simulink library "inp"  (Simulink 8.2)
settings: Solver=ode45  SolverMode=SingleTasking  StartTime=0.0  StopTime=10.0  RelTol=1e-3
undefined symbols (not defined in the file or in its folder): C_TICK_TIME_S, ENUM_FAULTSTATE_FAIL, ENUM_FAULTSTATE_INDETERMINATE, ENUM_FAULTSTATE_PASS, ENUM_KEYSWITCH_ON, FALSE, KINP_ABS1CANCommuOk_flg_ovrdflg, KINP_ABS1CANCommuOk_flg_ovrdval, KINP_ABS1CANPeriodTime_s, KINP_ABS1CANRolCntOk_flg_ovrdflg, KINP_ABS1CANRolCntOk_flg_ovrdval, KINP_ABS2CANChkSumOk_flg_ovrdflg, KINP_ABS2CANChkSumOk_flg_ovrdval, KINP_ABS2CANCommuOk_flg_ovrdflg, KINP_ABS2CANCommuOk_flg_ovrdval, KINP_ABS2CANPeriodTime_s, KINP_ABS2CANRolCntOk_flg_ovrdflg, KINP_ABS2CANRolCntOk_flg_ovrdval, KINP_ABS3CANCommuOk_flg_ovrdflg, KINP_ABS3CANCommuOk_flg_ovrdval, KINP_ABS4CANCommuOk_flg_ovrdflg, KINP_ABS4CANCommuOk_flg_ovrdval, KINP_ABSActive_flg_defval, KINP_ABSActive_flg_invalid, KINP_ABSActive_flg_ovrdflg, KINP_ABSActive_flg_ovrdval, KINP_ABSActive_flg_setvalid, KINP_ABSActive_flg_srvcflg, KINP_ABSActive_flg_srvcval, KINP_ABSCANAlive_flg_ovrdflg, KINP_ABSCANAlive_flg_ovrdval, KINP_ABSCANCommuOk_flg_ovrdflg, KINP_ABSCANCommuOk_flg_ovrdval, KINP_ABSCANValidOk_flg_ovrdflg, KINP_ABSCANValidOk_flg_ovrdval, KINP_ABSFault_flg_defval, KINP_ABSFault_flg_invalid, KINP_ABSFault_flg_ovrdflg, KINP_ABSFault_flg_ovrdval, KINP_ABSFault_flg_setvalid, KINP_ABSFault_flg_srvcflg, KINP_ABSFault_flg_srvcval, KINP_AC1CANCommuOk_flg_ovrdflg, KINP_AC1CANCommuOk_flg_ovrdval, KINP_ACCANAlive_flg_ovrdflg, KINP_ACCANAlive_flg_ovrdval, KINP_ACCANCommuOk_flg_ovrdflg, KINP_ACCANCommuOk_flg_ovrdval, KINP_ACCANValidOk_flg_ovrdflg, KINP_ACCANValidOk_flg_ovrdval, KINP_ACCCancelH_flg_defval, KINP_ACCCancelH_flg_invalid, KINP_ACCCancelH_flg_ovrdflg, KINP_ACCCancelH_flg_ovrdval, KINP_ACCCancelH_flg_setvalid, KINP_ACCCancelH_flg_srvcflg, KINP_ACCCancelH_flg_srvcval, KINP_AccelPedalPosn_pct_defval, KINP_AccelPedalPosn_pct_gain, KINP_AccelPedalPosn_pct_invalid ... (+1866)
libraries: inp x192, hcu_lib x34, simulink x33
(part 11 of 21)

== system inp/INP/EPM_signals/Cam_Obstacle_8 ==
  BHAL_outputs : Inport
  CANvalid_bus : Inport  Port=2
  Bus Creator : BusCreator  Inputs=40
  CANVBusSel{141..150} (10 alike) : BusSelector  OutputSignals=VINP_CANMsgValidEPM_flg.VINP_CANMsgValidEPM27_flg
  CANVBusSel{151..155} (5 alike) : BusSelector  OutputSignals=VINP_CANMsgValidEPM_flg.VINP_CANMsgValidEPM28_flg
  CANVBusSel{156..160} (5 alike) : BusSelector  OutputSignals=VINP_CANMsgValidEPM_flg.VINP_CANMsgValidEPM29_flg
  Constant{141..160} (20 alike) : Constant  Value=TRUE
  Conv{141,145..149,153..154} (8 alike) : DataTypeConversion  OutDataTypeStr=uint8
  Conv{142..144,151..152,156..159} (9 alike) : DataTypeConversion  OutDataTypeStr=single
  Conv150 : DataTypeConversion  OutDataTypeStr=boolean
  Conv155 : DataTypeConversion  OutDataTypeStr=boolean
  Conv160 : DataTypeConversion  OutDataTypeStr=boolean
  HalBusSel141 : BusSelector  OutputSignals=VHAL_CamObs8ID_num
  HalBusSel142 : BusSelector  OutputSignals=VHAL_CamObs8PosX_m
  HalBusSel143 : BusSelector  OutputSignals=VHAL_CamObs8PosY_m
  HalBusSel144 : BusSelector  OutputSignals=VHAL_CamObs8VelX_mps
  HalBusSel145 : BusSelector  OutputSignals=VHAL_CamObs8Type_enum
  HalBusSel146 : BusSelector  OutputSignals=VHAL_CamObs8Status_enum
  HalBusSel147 : BusSelector  OutputSignals=VHAL_CamObs8Valid_enum
  HalBusSel148 : BusSelector  OutputSignals=VHAL_CamObs8MoveInO_enum
  HalBusSel149 : BusSelector  OutputSignals=VHAL_CamObs8Blinker_enum
  HalBusSel150 : BusSelector  OutputSignals=VHAL_CamObs8BrakeLight_flg
  HalBusSel151 : BusSelector  OutputSignals=VHAL_CamObs8Length_m
  HalBusSel152 : BusSelector  OutputSignals=VHAL_CamObs8Width_m
  HalBusSel153 : BusSelector  OutputSignals=VHAL_CamObs8Age_cnt
  HalBusSel154 : BusSelector  OutputSignals=VHAL_CamObs8Lane_enum
  HalBusSel155 : BusSelector  OutputSignals=VHAL_CamObs8CIPV_flg
  HalBusSel156 : BusSelector  OutputSignals=VHAL_CamObs8VelY_mps
  HalBusSel157 : BusSelector  OutputSignals=VHAL_CamObs8AccX_mps2
  HalBusSel158 : BusSelector  OutputSignals=VHAL_CamObs8Ang_deg
  HalBusSel159 : BusSelector  OutputSignals=VHAL_CamObs8AngRate_degps
  HalBusSel160 : BusSelector  OutputSignals=VHAL_CamObs8Replaced_flg
  Term{141..160} (20 alike) : Terminator
  Cam_Obstacle_8 : Outport
  wires:
  [BHAL_outputs] -> HalBusSel141/1
  HalBusSel141/1 -> Conv141/1  "<VHAL_CamObs8ID_num>"
  [CANvalid_bus] -> CANVBusSel141/1
  Conv141/1 -> Bus Creator/1  "VINP_CamObs8ID_num_InHAL"
  Constant141/1 -> Bus Creator/2  "VINP_CamObs8IDValid_flg_InHAL"
  CANVBusSel141/1 -> Term141/1  "<VINP_CANMsgValidEPM27_flg>"
  [BHAL_outputs] -> HalBusSel142/1
  HalBusSel142/1 -> Conv142/1  "<VHAL_CamObs8PosX_m>"
  [CANvalid_bus] -> CANVBusSel142/1
  Conv142/1 -> Bus Creator/3  "VINP_CamObs8PosX_m_InHAL"
  Constant142/1 -> Bus Creator/4  "VINP_CamObs8PosXValid_flg_InHAL"
  CANVBusSel142/1 -> Term142/1  "<VINP_CANMsgValidEPM27_flg>"
  [BHAL_outputs] -> HalBusSel143/1
  HalBusSel143/1 -> Conv143/1  "<VHAL_CamObs8PosY_m>"
  [CANvalid_bus] -> CANVBusSel143/1
  Conv143/1 -> Bus Creator/5  "VINP_CamObs8PosY_m_InHAL"
  Constant143/1 -> Bus Creator/6  "VINP_CamObs8PosYValid_flg_InHAL"
  CANVBusSel143/1 -> Term143/1  "<VINP_CANMsgValidEPM27_flg>"
  [BHAL_outputs] -> HalBusSel144/1
  HalBusSel144/1 -> Conv144/1  "<VHAL_CamObs8VelX_mps>"
  [CANvalid_bus] -> CANVBusSel144/1
  Conv144/1 -> Bus Creator/7  "VINP_CamObs8VelX_mps_InHAL"
  Constant144/1 -> Bus Creator/8  "VINP_CamObs8VelXValid_flg_InHAL"
  CANVBusSel144/1 -> Term144/1  "<VINP_CANMsgValidEPM27_flg>"
  [BHAL_outputs] -> HalBusSel145/1
  HalBusSel145/1 -> Conv145/1  "<VHAL_CamObs8Type_enum>"
  [CANvalid_bus] -> CANVBusSel145/1
  Conv145/1 -> Bus Creator/9  "VINP_CamObs8Type_enum_InHAL"
  Constant145/1 -> Bus Creator/10  "VINP_CamObs8TypeValid_flg_InHAL"
  CANVBusSel145/1 -> Term145/1  "<VINP_CANMsgValidEPM27_flg>"
  [BHAL_outputs] -> HalBusSel146/1
  Conv146/1 -> Bus Creator/11  "VINP_CamObs8Status_enum_InHAL"
  HalBusSel146/1 -> Conv146/1  "<VHAL_CamObs8Status_enum>"
  [CANvalid_bus] -> CANVBusSel146/1
  Constant146/1 -> Bus Creator/12  "VINP_CamObs8StatusValid_flg_InHAL"
  CANVBusSel146/1 -> Term146/1  "<VINP_CANMsgValidEPM27_flg>"
  [BHAL_outputs] -> HalBusSel147/1
  [CANvalid_bus] -> CANVBusSel147/1
  HalBusSel147/1 -> Conv147/1  "<VHAL_CamObs8Valid_enum>"
  Conv147/1 -> Bus Creator/13  "VINP_CamObs8Valid_enum_InHAL"
  Constant147/1 -> Bus Creator/14  "VINP_CamObs8ValidValid_flg_InHAL"
  CANVBusSel147/1 -> Term147/1  "<VINP_CANMsgValidEPM27_flg>"
  [BHAL_outputs] -> HalBusSel148/1
  Conv148/1 -> Bus Creator/15  "VINP_CamObs8MoveInO_enum_InHAL"
  HalBusSel148/1 -> Conv148/1  "<VHAL_CamObs8MoveInO_enum>"
  [CANvalid_bus] -> CANVBusSel148/1
  Constant148/1 -> Bus Creator/16  "VINP_CamObs8MoveInOValid_flg_InHAL"
  CANVBusSel148/1 -> Term148/1  "<VINP_CANMsgValidEPM27_flg>"
  [BHAL_outputs] -> HalBusSel149/1
  Conv149/1 -> Bus Creator/17  "VINP_CamObs8Blinker_enum_InHAL"
  HalBusSel149/1 -> Conv149/1  "<VHAL_CamObs8Blinker_enum>"
  [CANvalid_bus] -> CANVBusSel149/1
  Constant149/1 -> Bus Creator/18  "VINP_CamObs8BlinkerValid_flg_InHAL"
  CANVBusSel149/1 -> Term149/1  "<VINP_CANMsgValidEPM27_flg>"
  [BHAL_outputs] -> HalBusSel150/1
  Conv150/1 -> Bus Creator/19  "VINP_CamObs8BrakeLight_flg_InHAL"
  HalBusSel150/1 -> Conv150/1  "<VHAL_CamObs8BrakeLight_flg>"
  [CANvalid_bus] -> CANVBusSel150/1
  Constant150/1 -> Bus Creator/20  "VINP_CamObs8BrakeLightValid_flg_InHAL"
  CANVBusSel150/1 -> Term150/1  "<VINP_CANMsgValidEPM27_flg>"
  [BHAL_outputs] -> HalBusSel151/1
  HalBusSel151/1 -> Conv151/1  "<VHAL_CamObs8Length_m>"
  [CANvalid_bus] -> CANVBusSel151/1
  Conv151/1 -> Bus Creator/21  "VINP_CamObs8Length_m_InHAL"
  Constant151/1 -> Bus Creator/22  "VINP_CamObs8LengthValid_flg_InHAL"
  CANVBusSel151/1 -> Term151/1  "<VINP_CANMsgValidEPM28_flg>"
  [BHAL_outputs] -> HalBusSel152/1
  HalBusSel152/1 -> Conv152/1  "<VHAL_CamObs8Width_m>"
  [CANvalid_bus] -> CANVBusSel152/1
  Conv152/1 -> Bus Creator/23  "VINP_CamObs8Width_m_InHAL"
  Constant152/1 -> Bus Creator/24  "VINP_CamObs8WidthValid_flg_InHAL"
  CANVBusSel152/1 -> Term152/1  "<VINP_CANMsgValidEPM28_flg>"
  [BHAL_outputs] -> HalBusSel153/1
  HalBusSel153/1 -> Conv153/1  "<VHAL_CamObs8Age_cnt>"
  [CANvalid_bus] -> CANVBusSel153/1
  Conv153/1 -> Bus Creator/25  "VINP_CamObs8Age_cnt_InHAL"
  Constant153/1 -> Bus Creator/26  "VINP_CamObs8AgeValid_flg_InHAL"
  CANVBusSel153/1 -> Term153/1  "<VINP_CANMsgValidEPM28_flg>"
  [BHAL_outputs] -> HalBusSel154/1
  HalBusSel154/1 -> Conv154/1  "<VHAL_CamObs8Lane_enum>"
  [CANvalid_bus] -> CANVBusSel154/1
  Conv154/1 -> Bus Creator/27  "VINP_CamObs8Lane_enum_InHAL"
  Constant154/1 -> Bus Creator/28  "VINP_CamObs8LaneValid_flg_InHAL"
  CANVBusSel154/1 -> Term154/1  "<VINP_CANMsgValidEPM28_flg>"
  [BHAL_outputs] -> HalBusSel155/1
  HalBusSel155/1 -> Conv155/1  "<VHAL_CamObs8CIPV_flg>"
  [CANvalid_bus] -> CANVBusSel155/1
  Conv155/1 -> Bus Creator/29  "VINP_CamObs8CIPV_flg_InHAL"
  Constant155/1 -> Bus Creator/30  "VINP_CamObs8CIPVValid_flg_InHAL"
  CANVBusSel155/1 -> Term155/1  "<VINP_CANMsgValidEPM28_flg>"
  [BHAL_outputs] -> HalBusSel156/1
  HalBusSel156/1 -> Conv156/1  "<VHAL_CamObs8VelY_mps>"
  [CANvalid_bus] -> CANVBusSel156/1
  Conv156/1 -> Bus Creator/31  "VINP_CamObs8VelY_mps_InHAL"
  Constant156/1 -> Bus Creator/32  "VINP_CamObs8VelYValid_flg_InHAL"
  CANVBusSel156/1 -> Term156/1  "<VINP_CANMsgValidEPM29_flg>"
  [BHAL_outputs] -> HalBusSel157/1
  HalBusSel157/1 -> Conv157/1  "<VHAL_CamObs8AccX_mps2>"
  [CANvalid_bus] -> CANVBusSel157/1
  Conv157/1 -> Bus Creator/33  "VINP_CamObs8AccX_mps2_InHAL"
  Constant157/1 -> Bus Creator/34  "VINP_CamObs8AccXValid_flg_InHAL"
  CANVBusSel157/1 -> Term157/1  "<VINP_CANMsgValidEPM29_flg>"
  [BHAL_outputs] -> HalBusSel158/1
  HalBusSel158/1 -> Conv158/1  "<VHAL_CamObs8Ang_deg>"
  [CANvalid_bus] -> CANVBusSel158/1
  Conv158/1 -> Bus Creator/35  "VINP_CamObs8Ang_deg_InHAL"
  Constant158/1 -> Bus Creator/36  "VINP_CamObs8AngValid_flg_InHAL"
  CANVBusSel158/1 -> Term158/1  "<VINP_CANMsgValidEPM29_flg>"
  [BHAL_outputs] -> HalBusSel159/1
  Conv159/1 -> Bus Creator/37  "VINP_CamObs8AngRate_degps_InHAL"
  HalBusSel159/1 -> Conv159/1  "<VHAL_CamObs8AngRate_degps>"
  [CANvalid_bus] -> CANVBusSel159/1
  Constant159/1 -> Bus Creator/38  "VINP_CamObs8AngRateValid_flg_InHAL"
  CANVBusSel159/1 -> Term159/1  "<VINP_CANMsgValidEPM29_flg>"
  [BHAL_outputs] -> HalBusSel160/1
  Conv160/1 -> Bus Creator/39  "VINP_CamObs8Replaced_flg_InHAL"
  HalBusSel160/1 -> Conv160/1  "<VHAL_CamObs8Replaced_flg>"
  [CANvalid_bus] -> CANVBusSel160/1
  Constant160/1 -> Bus Creator/40  "VINP_CamObs8ReplacedValid_flg_InHAL"
  CANVBusSel160/1 -> Term160/1  "<VINP_CANMsgValidEPM29_flg>"
  BHAL_outputs/1 -> [BHAL_outputs]
  CANvalid_bus/1 -> [CANvalid_bus]
  Bus Creator/1 -> Cam_Obstacle_8/1  "VINP_Obstacle8_BUS_InHAL"

== system inp/INP/EPM_signals/Cam_Obstacle_9 ==
  BHAL_outputs : Inport
  CANvalid_bus : Inport  Port=2
  Bus Creator : BusCreator  Inputs=40
  CANVBusSel{161..170} (10 alike) : BusSelector  OutputSignals=VINP_CANMsgValidEPM_flg.VINP_CANMsgValidEPM30_flg
  CANVBusSel{171..175} (5 alike) : BusSelector  OutputSignals=VINP_CANMsgValidEPM_flg.VINP_CANMsgValidEPM31_flg
  CANVBusSel{176..180} (5 alike) : BusSelector  OutputSignals=VINP_CANMsgValidEPM_flg.VINP_CANMsgValidEPM32_flg
  Constant{161..180} (20 alike) : Constant  Value=TRUE
  Conv{161,165..169,173..174} (8 alike) : DataTypeConversion  OutDataTypeStr=uint8
  Conv{162..164,171..172,176..179} (9 alike) : DataTypeConversion  OutDataTypeStr=single
  Conv170 : DataTypeConversion  OutDataTypeStr=boolean
  Conv175 : DataTypeConversion  OutDataTypeStr=boolean
  Conv180 : DataTypeConversion  OutDataTypeStr=boolean
  HalBusSel161 : BusSelector  OutputSignals=VHAL_CamObs9ID_num
  HalBusSel162 : BusSelector  OutputSignals=VHAL_CamObs9PosX_m
  HalBusSel163 : BusSelector  OutputSignals=VHAL_CamObs9PosY_m
  HalBusSel164 : BusSelector  OutputSignals=VHAL_CamObs9VelX_mps
  HalBusSel165 : BusSelector  OutputSignals=VHAL_CamObs9Type_enum
  HalBusSel166 : BusSelector  OutputSignals=VHAL_CamObs9Status_enum
  HalBusSel167 : BusSelector  OutputSignals=VHAL_CamObs9Valid_enum
  HalBusSel168 : BusSelector  OutputSignals=VHAL_CamObs9MoveInO_enum
  HalBusSel169 : BusSelector  OutputSignals=VHAL_CamObs9Blinker_enum
  HalBusSel170 : BusSelector  OutputSignals=VHAL_CamObs9BrakeLight_flg
  HalBusSel171 : BusSelector  OutputSignals=VHAL_CamObs9Length_m
  HalBusSel172 : BusSelector  OutputSignals=VHAL_CamObs9Width_m
  HalBusSel173 : BusSelector  OutputSignals=VHAL_CamObs9Age_cnt
  HalBusSel174 : BusSelector  OutputSignals=VHAL_CamObs9Lane_enum
  HalBusSel175 : BusSelector  OutputSignals=VHAL_CamObs9CIPV_flg
  HalBusSel176 : BusSelector  OutputSignals=VHAL_CamObs9VelY_mps
  HalBusSel177 : BusSelector  OutputSignals=VHAL_CamObs9AccX_mps2
  HalBusSel178 : BusSelector  OutputSignals=VHAL_CamObs9Ang_deg
  HalBusSel179 : BusSelector  OutputSignals=VHAL_CamObs9AngRate_degps
  HalBusSel180 : BusSelector  OutputSignals=VHAL_CamObs9Replaced_flg
  Term{161..180} (20 alike) : Terminator
  Cam_Obstacle_9 : Outport
  wires:
  [BHAL_outputs] -> HalBusSel161/1
  HalBusSel161/1 -> Conv161/1  "<VHAL_CamObs9ID_num>"
  [CANvalid_bus] -> CANVBusSel161/1
  Conv161/1 -> Bus Creator/1  "VINP_CamObs9ID_num_InHAL"
  Constant161/1 -> Bus Creator/2  "VINP_CamObs9IDValid_flg_InHAL"
  CANVBusSel161/1 -> Term161/1  "<VINP_CANMsgValidEPM30_flg>"
  [BHAL_outputs] -> HalBusSel162/1
  HalBusSel162/1 -> Conv162/1  "<VHAL_CamObs9PosX_m>"
  [CANvalid_bus] -> CANVBusSel162/1
  Conv162/1 -> Bus Creator/3  "VINP_CamObs9PosX_m_InHAL"
  Constant162/1 -> Bus Creator/4  "VINP_CamObs9PosXValid_flg_InHAL"
  CANVBusSel162/1 -> Term162/1  "<VINP_CANMsgValidEPM30_flg>"
  [BHAL_outputs] -> HalBusSel163/1
  HalBusSel163/1 -> Conv163/1  "<VHAL_CamObs9PosY_m>"
  [CANvalid_bus] -> CANVBusSel163/1
  Conv163/1 -> Bus Creator/5  "VINP_CamObs9PosY_m_InHAL"
  Constant163/1 -> Bus Creator/6  "VINP_CamObs9PosYValid_flg_InHAL"
  CANVBusSel163/1 -> Term163/1  "<VINP_CANMsgValidEPM30_flg>"
  [BHAL_outputs] -> HalBusSel164/1
  HalBusSel164/1 -> Conv164/1  "<VHAL_CamObs9VelX_mps>"
  [CANvalid_bus] -> CANVBusSel164/1
  Conv164/1 -> Bus Creator/7  "VINP_CamObs9VelX_mps_InHAL"
  Constant164/1 -> Bus Creator/8  "VINP_CamObs9VelXValid_flg_InHAL"
  CANVBusSel164/1 -> Term164/1  "<VINP_CANMsgValidEPM30_flg>"
  [BHAL_outputs] -> HalBusSel165/1
  HalBusSel165/1 -> Conv165/1  "<VHAL_CamObs9Type_enum>"
  [CANvalid_bus] -> CANVBusSel165/1
  Conv165/1 -> Bus Creator/9  "VINP_CamObs9Type_enum_InHAL"
  Constant165/1 -> Bus Creator/10  "VINP_CamObs9TypeValid_flg_InHAL"
  CANVBusSel165/1 -> Term165/1  "<VINP_CANMsgValidEPM30_flg>"
  [BHAL_outputs] -> HalBusSel166/1
  Conv166/1 -> Bus Creator/11  "VINP_CamObs9Status_enum_InHAL"
  HalBusSel166/1 -> Conv166/1  "<VHAL_CamObs9Status_enum>"
  [CANvalid_bus] -> CANVBusSel166/1
  Constant166/1 -> Bus Creator/12  "VINP_CamObs9StatusValid_flg_InHAL"
  CANVBusSel166/1 -> Term166/1  "<VINP_CANMsgValidEPM30_flg>"
  [BHAL_outputs] -> HalBusSel167/1
  [CANvalid_bus] -> CANVBusSel167/1
  HalBusSel167/1 -> Conv167/1  "<VHAL_CamObs9Valid_enum>"
  Conv167/1 -> Bus Creator/13  "VINP_CamObs9Valid_enum_InHAL"
  Constant167/1 -> Bus Creator/14  "VINP_CamObs9ValidValid_flg_InHAL"
  CANVBusSel167/1 -> Term167/1  "<VINP_CANMsgValidEPM30_flg>"
  [BHAL_outputs] -> HalBusSel168/1
  Conv168/1 -> Bus Creator/15  "VINP_CamObs9MoveInO_enum_InHAL"
  HalBusSel168/1 -> Conv168/1  "<VHAL_CamObs9MoveInO_enum>"
  [CANvalid_bus] -> CANVBusSel168/1
  Constant168/1 -> Bus Creator/16  "VINP_CamObs9MoveInOValid_flg_InHAL"
  CANVBusSel168/1 -> Term168/1  "<VINP_CANMsgValidEPM30_flg>"
  [BHAL_outputs] -> HalBusSel169/1
  Conv169/1 -> Bus Creator/17  "VINP_CamObs9Blinker_enum_InHAL"
  HalBusSel169/1 -> Conv169/1  "<VHAL_CamObs9Blinker_enum>"
  [CANvalid_bus] -> CANVBusSel169/1
  Constant169/1 -> Bus Creator/18  "VINP_CamObs9BlinkerValid_flg_InHAL"
  CANVBusSel169/1 -> Term169/1  "<VINP_CANMsgValidEPM30_flg>"
  [BHAL_outputs] -> HalBusSel170/1
  Conv170/1 -> Bus Creator/19  "VINP_CamObs9BrakeLight_flg_InHAL"
  HalBusSel170/1 -> Conv170/1  "<VHAL_CamObs9BrakeLight_flg>"
  [CANvalid_bus] -> CANVBusSel170/1
  Constant170/1 -> Bus Creator/20  "VINP_CamObs9BrakeLightValid_flg_InHAL"
  CANVBusSel170/1 -> Term170/1  "<VINP_CANMsgValidEPM30_flg>"
  [BHAL_outputs] -> HalBusSel171/1
  HalBusSel171/1 -> Conv171/1  "<VHAL_CamObs9Length_m>"
  [CANvalid_bus] -> CANVBusSel171/1
  Conv171/1 -> Bus Creator/21  "VINP_CamObs9Length_m_InHAL"
  Constant171/1 -> Bus Creator/22  "VINP_CamObs9LengthValid_flg_InHAL"
  CANVBusSel171/1 -> Term171/1  "<VINP_CANMsgValidEPM31_flg>"
  [BHAL_outputs] -> HalBusSel172/1
  HalBusSel172/1 -> Conv172/1  "<VHAL_CamObs9Width_m>"
  [CANvalid_bus] -> CANVBusSel172/1
  Conv172/1 -> Bus Creator/23  "VINP_CamObs9Width_m_InHAL"
  Constant172/1 -> Bus Creator/24  "VINP_CamObs9WidthValid_flg_InHAL"
  CANVBusSel172/1 -> Term172/1  "<VINP_CANMsgValidEPM31_flg>"
  [BHAL_outputs] -> HalBusSel173/1
  HalBusSel173/1 -> Conv173/1  "<VHAL_CamObs9Age_cnt>"
  [CANvalid_bus] -> CANVBusSel173/1
  Conv173/1 -> Bus Creator/25  "VINP_CamObs9Age_cnt_InHAL"
  Constant173/1 -> Bus Creator/26  "VINP_CamObs9AgeValid_flg_InHAL"
  CANVBusSel173/1 -> Term173/1  "<VINP_CANMsgValidEPM31_flg>"
  [BHAL_outputs] -> HalBusSel174/1
  HalBusSel174/1 -> Conv174/1  "<VHAL_CamObs9Lane_enum>"
  [CANvalid_bus] -> CANVBusSel174/1
  Conv174/1 -> Bus Creator/27  "VINP_CamObs9Lane_enum_InHAL"
  Constant174/1 -> Bus Creator/28  "VINP_CamObs9LaneValid_flg_InHAL"
  CANVBusSel174/1 -> Term174/1  "<VINP_CANMsgValidEPM31_flg>"
  [BHAL_outputs] -> HalBusSel175/1
  HalBusSel175/1 -> Conv175/1  "<VHAL_CamObs9CIPV_flg>"
  [CANvalid_bus] -> CANVBusSel175/1
  Conv175/1 -> Bus Creator/29  "VINP_CamObs9CIPV_flg_InHAL"
  Constant175/1 -> Bus Creator/30  "VINP_CamObs9CIPVValid_flg_InHAL"
  CANVBusSel175/1 -> Term175/1  "<VINP_CANMsgValidEPM31_flg>"
  [BHAL_outputs] -> HalBusSel176/1
  HalBusSel176/1 -> Conv176/1  "<VHAL_CamObs9VelY_mps>"
  [CANvalid_bus] -> CANVBusSel176/1
  Conv176/1 -> Bus Creator/31  "VINP_CamObs9VelY_mps_InHAL"
  Constant176/1 -> Bus Creator/32  "VINP_CamObs9VelYValid_flg_InHAL"
  CANVBusSel176/1 -> Term176/1  "<VINP_CANMsgValidEPM32_flg>"
  [BHAL_outputs] -> HalBusSel177/1
  HalBusSel177/1 -> Conv177/1  "<VHAL_CamObs9AccX_mps2>"
  [CANvalid_bus] -> CANVBusSel177/1
  Conv177/1 -> Bus Creator/33  "VINP_CamObs9AccX_mps2_InHAL"
  Constant177/1 -> Bus Creator/34  "VINP_CamObs9AccXValid_flg_InHAL"
  CANVBusSel177/1 -> Term177/1  "<VINP_CANMsgValidEPM32_flg>"
  [BHAL_outputs] -> HalBusSel178/1
  HalBusSel178/1 -> Conv178/1  "<VHAL_CamObs9Ang_deg>"
  [CANvalid_bus] -> CANVBusSel178/1
  Conv178/1 -> Bus Creator/35  "VINP_CamObs9Ang_deg_InHAL"
  Constant178/1 -> Bus Creator/36  "VINP_CamObs9AngValid_flg_InHAL"
  CANVBusSel178/1 -> Term178/1  "<VINP_CANMsgValidEPM32_flg>"
  [BHAL_outputs] -> HalBusSel179/1
  Conv179/1 -> Bus Creator/37  "VINP_CamObs9AngRate_degps_InHAL"
  HalBusSel179/1 -> Conv179/1  "<VHAL_CamObs9AngRate_degps>"
  [CANvalid_bus] -> CANVBusSel179/1
  Constant179/1 -> Bus Creator/38  "VINP_CamObs9AngRateValid_flg_InHAL"
  CANVBusSel179/1 -> Term179/1  "<VINP_CANMsgValidEPM32_flg>"
  [BHAL_outputs] -> HalBusSel180/1
  Conv180/1 -> Bus Creator/39  "VINP_CamObs9Replaced_flg_InHAL"
  HalBusSel180/1 -> Conv180/1  "<VHAL_CamObs9Replaced_flg>"
  [CANvalid_bus] -> CANVBusSel180/1
  Constant180/1 -> Bus Creator/40  "VINP_CamObs9ReplacedValid_flg_InHAL"
  CANVBusSel180/1 -> Term180/1  "<VINP_CANMsgValidEPM32_flg>"
  BHAL_outputs/1 -> [BHAL_outputs]
  CANvalid_bus/1 -> [CANvalid_bus]
  Bus Creator/1 -> Cam_Obstacle_9/1  "VINP_Obstacle9_BUS_InHAL"

== system inp/INP/EPM_signals/Cam_Right_Next_Lane ==
  BHAL_outputs : Inport
  CANvalid_bus : Inport  Port=2
  Bus Creator : BusCreator  Inputs=18
  CANVBusSel{1..9} (9 alike) : BusSelector  OutputSignals=VINP_CANMsgValidEPM_flg.VINP_CANMsgValidEPM1_flg
  Constant{1..9} (9 alike) : Constant  Value=TRUE
  Conv{1..4,7..9} (7 alike) : DataTypeConversion  OutDataTypeStr=single
  Conv5 : DataTypeConversion  OutDataTypeStr=uint8
  Conv6 : DataTypeConversion  OutDataTypeStr=uint8
  Gain{1..4} (4 alike) : Gain  Gain=-1
  HalBusSel1 : BusSelector  OutputSignals=VHAL_NextLaneC0R_m
  HalBusSel2 : BusSelector  OutputSignals=VHAL_NextLaneC1R_rad
  HalBusSel3 : BusSelector  OutputSignals=VHAL_NextLaneC2R_rat
  HalBusSel4 : BusSelector  OutputSignals=VHAL_NextLaneC3R_rat
  HalBusSel5 : BusSelector  OutputSignals=VHAL_NextLaneQualityR_enum
  HalBusSel6 : BusSelector  OutputSignals=VHAL_NextLaneTypeR_enum
  HalBusSel7 : BusSelector  OutputSignals=VHAL_NextLaneMrkWidR_m
  HalBusSel8 : BusSelector  OutputSignals=VHAL_NextViewRangeStartR_m
  HalBusSel9 : BusSelector  OutputSignals=VHAL_NextViewRangeEndR_m
  Term{1..9} (9 alike) : Terminator
  VINP_RightNextLane_BUS_InHAL : Outport
  wires:
  [CANvalid_bus] -> CANVBusSel9/1
  CANVBusSel9/1 -> Term9/1  "<VINP_CANMsgValidEPM1_flg>"
  [CANvalid_bus] -> CANVBusSel8/1
  CANVBusSel8/1 -> Term8/1  "<VINP_CANMsgValidEPM1_flg>"
  [CANvalid_bus] -> CANVBusSel7/1
  CANVBusSel7/1 -> Term7/1  "<VINP_CANMsgValidEPM1_flg>"
  [CANvalid_bus] -> CANVBusSel6/1
  CANVBusSel6/1 -> Term6/1  "<VINP_CANMsgValidEPM1_flg>"
  [CANvalid_bus] -> CANVBusSel5/1
  CANVBusSel5/1 -> Term5/1  "<VINP_CANMsgValidEPM1_flg>"
  [CANvalid_bus] -> CANVBusSel4/1
  CANVBusSel4/1 -> Term4/1  "<VINP_CANMsgValidEPM1_flg>"
  [CANvalid_bus] -> CANVBusSel3/1
  CANVBusSel3/1 -> Term3/1  "<VINP_CANMsgValidEPM1_flg>"
  [CANvalid_bus] -> CANVBusSel2/1
  CANVBusSel2/1 -> Term2/1  "<VINP_CANMsgValidEPM1_flg>"
  [BHAL_outputs] -> HalBusSel1/1
  HalBusSel1/1 -> Gain3/1  "<VHAL_NextLaneC0R_m>"
  [CANvalid_bus] -> CANVBusSel1/1
  Constant1/1 -> Bus Creator/2  "VINP_NextLaneC0RValid_flg_InHAL"
  Conv1/1 -> Bus Creator/1  "VINP_NextLaneC0R_m_InHAL"
  CANVBusSel1/1 -> Term1/1  "<VINP_CANMsgValidEPM1_flg>"
  [BHAL_outputs] -> HalBusSel2/1
  HalBusSel2/1 -> Gain1/1  "<VHAL_NextLaneC1R_rad>"
  Constant2/1 -> Bus Creator/4  "VINP_NextLaneC1RValid_flg_InHAL"
  Conv2/1 -> Bus Creator/3  "VINP_NextLaneC1R_rad_InHAL"
  HalBusSel3/1 -> Gain2/1  "<VHAL_NextLaneC2R_rat>"
  [BHAL_outputs] -> HalBusSel3/1
  Constant3/1 -> Bus Creator/6  "VINP_NextLaneC2RValid_flg_InHAL"
  Conv3/1 -> Bus Creator/5  "VINP_NextLaneC2R_rat_InHAL"
  HalBusSel4/1 -> Gain4/1  "<VHAL_NextLaneC3R_rat>"
  [BHAL_outputs] -> HalBusSel4/1
  Constant4/1 -> Bus Creator/8  "VINP_NextLaneC3RValid_flg_InHAL"
  Conv4/1 -> Bus Creator/7  "VINP_NextLaneC3R_rat_InHAL"
  HalBusSel5/1 -> Conv5/1  "<VHAL_NextLaneQualityR_enum>"
  [BHAL_outputs] -> HalBusSel5/1
  Constant5/1 -> Bus Creator/10  "VINP_NextLaneQualityRValid_flg_InHAL"
  Conv5/1 -> Bus Creator/9  "VINP_NextLaneQualityR_enum_InHAL"
  [BHAL_outputs] -> HalBusSel6/1
  HalBusSel6/1 -> Conv6/1  "<VHAL_NextLaneTypeR_enum>"
  Conv6/1 -> Bus Creator/11  "VINP_NextLaneTypeR_enum_InHAL"
  Constant6/1 -> Bus Creator/12  "VINP_NextLaneTypeRValid_flg_InHAL"
  [BHAL_outputs] -> HalBusSel7/1
  HalBusSel7/1 -> Conv7/1  "<VHAL_NextLaneMrkWidR_m>"
  Constant7/1 -> Bus Creator/14  "VINP_NextLaneMrkWidRValid_flg_InHAL"
  Conv7/1 -> Bus Creator/13  "VINP_NextLaneMrkWidR_m_InHAL"
  [BHAL_outputs] -> HalBusSel8/1
  HalBusSel8/1 -> Conv8/1  "<VHAL_NextViewRangeStartR_m>"
  Conv8/1 -> Bus Creator/15  "VINP_NextViewRangeStartR_m_InHAL"
  Constant8/1 -> Bus Creator/16  "VINP_NextViewRangeStartRValid_flg_InHAL"
  [BHAL_outputs] -> HalBusSel9/1
  HalBusSel9/1 -> Conv9/1  "<VHAL_NextViewRangeEndR_m>"
  Conv9/1 -> Bus Creator/17  "VINP_NextViewRangeEndR_m_InHAL"
  Constant9/1 -> Bus Creator/18  "VINP_NextViewRangeEndRValid_flg_InHAL"
  BHAL_outputs/1 -> [BHAL_outputs]
  CANvalid_bus/1 -> [CANvalid_bus]
  Bus Creator/1 -> VINP_RightNextLane_BUS_InHAL/1  "VINP_RightNextLane_BUS_InHAL"
  Gain3/1 -> Conv1/1
  Gain1/1 -> Conv2/1
  Gain2/1 -> Conv3/1
  Gain4/1 -> Conv4/1

== system inp/INP/EPM_signals/LaneC0Left ==
  signal_in : Inport
  msg_valid : Inport  Port=2
  Active_fault_flg : Inport  Port=3
  can_signal_process_wio_validity : inp/can_signal_process_wio_validity
  conv_gain_offset : Subsystem
  defval : Constant  Value=KINP_LaneC0L_m_defval
  invalid : Constant  Value=KINP_LaneC0L_m_invalid
  min_max_limit : Subsystem
  ovrdflg : Constant  Value=KINP_LaneC0L_m_ovrdflg
  ovrdval : Constant  Value=KINP_LaneC0L_m_ovrdval
  setvalid : Constant  Value=KINP_LaneC0L_m_setvalid
  srvcflg : Constant  Value=KINP_LaneC0L_m_srvcflg
  srvcval : Constant  Value=KINP_LaneC0L_m_srvcval
  signal_out : Outport
  signal_valid_out : Outport  Port=2
  wires:
  can_signal_process_wio_validity/1 -> min_max_limit/1(in)
  can_signal_process_wio_validity/2 -> signal_valid_out/1
  signal_in/1 -> conv_gain_offset/1(in)
  msg_valid/1 -> can_signal_process_wio_validity/2
  defval/1 -> can_signal_process_wio_validity/4
  invalid/1 -> can_signal_process_wio_validity/5
  conv_gain_offset/1(out) -> can_signal_process_wio_validity/1
  min_max_limit/1(out) -> signal_out/1
  setvalid/1 -> can_signal_process_wio_validity/6
  ovrdflg/1 -> can_signal_process_wio_validity/7
  ovrdval/1 -> can_signal_process_wio_validity/8
  srvcflg/1 -> can_signal_process_wio_validity/9
  srvcval/1 -> can_signal_process_wio_validity/10
  Active_fault_flg/1 -> can_signal_process_wio_validity/3

== system inp/INP/EPM_signals/LaneC0Right ==
  signal_in : Inport
  msg_valid : Inport  Port=2
  Active_fault_flg : Inport  Port=3
  can_signal_process_wio_validity : inp/can_signal_process_wio_validity
  conv_gain_offset : Subsystem
  defval : Constant  Value=KINP_LaneC0R_m_defval
  invalid : Constant  Value=KINP_LaneC0R_m_invalid
  min_max_limit : Subsystem
  ovrdflg : Constant  Value=KINP_LaneC0R_m_ovrdflg
  ovrdval : Constant  Value=KINP_LaneC0R_m_ovrdval
  setvalid : Constant  Value=KINP_LaneC0R_m_setvalid
  srvcflg : Constant  Value=KINP_LaneC0R_m_srvcflg
  srvcval : Constant  Value=KINP_LaneC0R_m_srvcval
  signal_out : Outport
  signal_valid_out : Outport  Port=2
  wires:
  can_signal_process_wio_validity/1 -> min_max_limit/1(in)
  can_signal_process_wio_validity/2 -> signal_valid_out/1
  signal_in/1 -> conv_gain_offset/1(in)
  msg_valid/1 -> can_signal_process_wio_validity/2
  defval/1 -> can_signal_process_wio_validity/4
  invalid/1 -> can_signal_process_wio_validity/5
  conv_gain_offset/1(out) -> can_signal_process_wio_validity/1
  min_max_limit/1(out) -> signal_out/1
  setvalid/1 -> can_signal_process_wio_validity/6
  ovrdflg/1 -> can_signal_process_wio_validity/7
  ovrdval/1 -> can_signal_process_wio_validity/8
  srvcflg/1 -> can_signal_process_wio_validity/9
  srvcval/1 -> can_signal_process_wio_validity/10
  Active_fault_flg/1 -> can_signal_process_wio_validity/3

== system inp/INP/EPM_signals/LaneC1Left ==
  signal_in : Inport
  msg_valid : Inport  Port=2
  Active_fault_flg : Inport  Port=3
  can_signal_process_wio_validity : inp/can_signal_process_wio_validity
  conv_gain_offset : Subsystem
  defval : Constant  Value=KINP_LaneC1L_rad_defval
  invalid : Constant  Value=KINP_LaneC1L_rad_invalid
  min_max_limit : Subsystem
  ovrdflg : Constant  Value=KINP_LaneC1L_rad_ovrdflg
  ovrdval : Constant  Value=KINP_LaneC1L_rad_ovrdval
  setvalid : Constant  Value=KINP_LaneC1L_rad_setvalid
  srvcflg : Constant  Value=KINP_LaneC1L_rad_srvcflg
  srvcval : Constant  Value=KINP_LaneC1L_rad_srvcval
  signal_out : Outport
  signal_valid_out : Outport  Port=2
  wires:
  Active_fault_flg/1 -> can_signal_process_wio_validity/3
  srvcval/1 -> can_signal_process_wio_validity/10
  srvcflg/1 -> can_signal_process_wio_validity/9
  ovrdval/1 -> can_signal_process_wio_validity/8
  ovrdflg/1 -> can_signal_process_wio_validity/7
  setvalid/1 -> can_signal_process_wio_validity/6
  min_max_limit/1(out) -> signal_out/1
  conv_gain_offset/1(out) -> can_signal_process_wio_validity/1
  invalid/1 -> can_signal_process_wio_validity/5
  defval/1 -> can_signal_process_wio_validity/4
  msg_valid/1 -> can_signal_process_wio_validity/2
  signal_in/1 -> conv_gain_offset/1(in)
  can_signal_process_wio_validity/2 -> signal_valid_out/1
  can_signal_process_wio_validity/1 -> min_max_limit/1(in)

== system inp/INP/EPM_signals/LaneC1Right ==
  signal_in : Inport
  msg_valid : Inport  Port=2
  Active_fault_flg : Inport  Port=3
  can_signal_process_wio_validity : inp/can_signal_process_wio_validity
  conv_gain_offset : Subsystem
  defval : Constant  Value=KINP_LaneC1R_rad_defval
  invalid : Constant  Value=KINP_LaneC1R_rad_invalid
  min_max_limit : Subsystem
  ovrdflg : Constant  Value=KINP_LaneC1R_rad_ovrdflg
  ovrdval : Constant  Value=KINP_LaneC1R_rad_ovrdval
  setvalid : Constant  Value=KINP_LaneC1R_rad_setvalid
  srvcflg : Constant  Value=KINP_LaneC1R_rad_srvcflg
  srvcval : Constant  Value=KINP_LaneC1R_rad_srvcval
  signal_out : Outport
  signal_valid_out : Outport  Port=2
  wires:
  Active_fault_flg/1 -> can_signal_process_wio_validity/3
  srvcval/1 -> can_signal_process_wio_validity/10
  srvcflg/1 -> can_signal_process_wio_validity/9
  ovrdval/1 -> can_signal_process_wio_validity/8
  ovrdflg/1 -> can_signal_process_wio_validity/7
  setvalid/1 -> can_signal_process_wio_validity/6
  min_max_limit/1(out) -> signal_out/1
  conv_gain_offset/1(out) -> can_signal_process_wio_validity/1
  invalid/1 -> can_signal_process_wio_validity/5
  defval/1 -> can_signal_process_wio_validity/4
  msg_valid/1 -> can_signal_process_wio_validity/2
  signal_in/1 -> conv_gain_offset/1(in)
  can_signal_process_wio_validity/2 -> signal_valid_out/1
  can_signal_process_wio_validity/1 -> min_max_limit/1(in)

== system inp/INP/EPM_signals/LaneC2Left ==
  signal_in : Inport
  msg_valid : Inport  Port=2
  Active_fault_flg : Inport  Port=3
  can_signal_process_wio_validity : inp/can_signal_process_wio_validity
  conv_gain_offset : Subsystem
  defval : Constant  Value=KINP_LaneC2L_rat_defval
  invalid : Constant  Value=KINP_LaneC2L_rat_invalid
  min_max_limit : Subsystem
  ovrdflg : Constant  Value=KINP_LaneC2L_rat_ovrdflg
  ovrdval : Constant  Value=KINP_LaneC2L_rat_ovrdval
  setvalid : Constant  Value=KINP_LaneC2L_rat_setvalid
  srvcflg : Constant  Value=KINP_LaneC2L_rat_srvcflg
  srvcval : Constant  Value=KINP_LaneC2L_rat_srvcval
  signal_out : Outport
  signal_valid_out : Outport  Port=2
  wires:
  can_signal_process_wio_validity/1 -> min_max_limit/1(in)
  can_signal_process_wio_validity/2 -> signal_valid_out/1
  signal_in/1 -> conv_gain_offset/1(in)
  msg_valid/1 -> can_signal_process_wio_validity/2
  defval/1 -> can_signal_process_wio_validity/4
  invalid/1 -> can_signal_process_wio_validity/5
  conv_gain_offset/1(out) -> can_signal_process_wio_validity/1
  min_max_limit/1(out) -> signal_out/1
  setvalid/1 -> can_signal_process_wio_validity/6
  ovrdflg/1 -> can_signal_process_wio_validity/7
  ovrdval/1 -> can_signal_process_wio_validity/8
  srvcflg/1 -> can_signal_process_wio_validity/9
  srvcval/1 -> can_signal_process_wio_validity/10
  Active_fault_flg/1 -> can_signal_process_wio_validity/3

== system inp/INP/EPM_signals/LaneC2Right ==
  signal_in : Inport
  msg_valid : Inport  Port=2
  Active_fault_flg : Inport  Port=3
  can_signal_process_wio_validity : inp/can_signal_process_wio_validity
  conv_gain_offset : Subsystem
  defval : Constant  Value=KINP_LaneC2R_rat_defval
  invalid : Constant  Value=KINP_LaneC2R_rat_invalid
  min_max_limit : Subsystem
  ovrdflg : Constant  Value=KINP_LaneC2R_rat_ovrdflg
  ovrdval : Constant  Value=KINP_LaneC2R_rat_ovrdval
  setvalid : Constant  Value=KINP_LaneC2R_rat_setvalid
  srvcflg : Constant  Value=KINP_LaneC2R_rat_srvcflg
  srvcval : Constant  Value=KINP_LaneC2R_rat_srvcval
  signal_out : Outport
  signal_valid_out : Outport  Port=2
  wires:
  can_signal_process_wio_validity/1 -> min_max_limit/1(in)
  can_signal_process_wio_validity/2 -> signal_valid_out/1
  signal_in/1 -> conv_gain_offset/1(in)
  msg_valid/1 -> can_signal_process_wio_validity/2
  defval/1 -> can_signal_process_wio_validity/4
  invalid/1 -> can_signal_process_wio_validity/5
  conv_gain_offset/1(out) -> can_signal_process_wio_validity/1
  min_max_limit/1(out) -> signal_out/1
  setvalid/1 -> can_signal_process_wio_validity/6
  ovrdflg/1 -> can_signal_process_wio_validity/7
  ovrdval/1 -> can_signal_process_wio_validity/8
  srvcflg/1 -> can_signal_process_wio_validity/9
  srvcval/1 -> can_signal_process_wio_validity/10
  Active_fault_flg/1 -> can_signal_process_wio_validity/3

== system inp/INP/EPM_signals/LaneC3Left ==
  signal_in : Inport
  msg_valid : Inport  Port=2
  Active_fault_flg : Inport  Port=3
  can_signal_process_wio_validity : inp/can_signal_process_wio_validity
  conv_gain_offset : Subsystem
  defval : Constant  Value=KINP_LaneC3L_rat_defval
  invalid : Constant  Value=KINP_LaneC3L_rat_invalid
  min_max_limit : Subsystem
  ovrdflg : Constant  Value=KINP_LaneC3L_rat_ovrdflg
  ovrdval : Constant  Value=KINP_LaneC3L_rat_ovrdval
  setvalid : Constant  Value=KINP_LaneC3L_rat_setvalid
  srvcflg : Constant  Value=KINP_LaneC3L_rat_srvcflg
  srvcval : Constant  Value=KINP_LaneC3L_rat_srvcval
  signal_out : Outport
  signal_valid_out : Outport  Port=2
  wires:
  Active_fault_flg/1 -> can_signal_process_wio_validity/3
  srvcval/1 -> can_signal_process_wio_validity/10
  srvcflg/1 -> can_signal_process_wio_validity/9
  ovrdval/1 -> can_signal_process_wio_validity/8
  ovrdflg/1 -> can_signal_process_wio_validity/7
  setvalid/1 -> can_signal_process_wio_validity/6
  min_max_limit/1(out) -> signal_out/1
  conv_gain_offset/1(out) -> can_signal_process_wio_validity/1
  invalid/1 -> can_signal_process_wio_validity/5
  defval/1 -> can_signal_process_wio_validity/4
  msg_valid/1 -> can_signal_process_wio_validity/2
  signal_in/1 -> conv_gain_offset/1(in)
  can_signal_process_wio_validity/2 -> signal_valid_out/1
  can_signal_process_wio_validity/1 -> min_max_limit/1(in)

== system inp/INP/EPM_signals/LaneC3Right ==
  signal_in : Inport
  msg_valid : Inport  Port=2
  Active_fault_flg : Inport  Port=3
  can_signal_process_wio_validity : inp/can_signal_process_wio_validity
  conv_gain_offset : Subsystem
  defval : Constant  Value=KINP_LaneC3R_rat_defval
  invalid : Constant  Value=KINP_LaneC3R_rat_invalid
  min_max_limit : Subsystem
  ovrdflg : Constant  Value=KINP_LaneC3R_rat_ovrdflg
  ovrdval : Constant  Value=KINP_LaneC3R_rat_ovrdval
  setvalid : Constant  Value=KINP_LaneC3R_rat_setvalid
  srvcflg : Constant  Value=KINP_LaneC3R_rat_srvcflg
  srvcval : Constant  Value=KINP_LaneC3R_rat_srvcval
  signal_out : Outport
  signal_valid_out : Outport  Port=2
  wires:
  Active_fault_flg/1 -> can_signal_process_wio_validity/3
  srvcval/1 -> can_signal_process_wio_validity/10
  srvcflg/1 -> can_signal_process_wio_validity/9
  ovrdval/1 -> can_signal_process_wio_validity/8
  ovrdflg/1 -> can_signal_process_wio_validity/7
  setvalid/1 -> can_signal_process_wio_validity/6
  min_max_limit/1(out) -> signal_out/1
  conv_gain_offset/1(out) -> can_signal_process_wio_validity/1
  invalid/1 -> can_signal_process_wio_validity/5
  defval/1 -> can_signal_process_wio_validity/4
  msg_valid/1 -> can_signal_process_wio_validity/2
  signal_in/1 -> conv_gain_offset/1(in)
  can_signal_process_wio_validity/2 -> signal_valid_out/1
  can_signal_process_wio_validity/1 -> min_max_limit/1(in)

== system inp/INP/EPM_signals/LaneCrossingLeft ==
  signal_in : Inport
  msg_valid : Inport  Port=2
  Active_fault_flg : Inport  Port=3
  can_signal_process_wio_validity : inp/can_signal_process_wio_validity
  defval : Constant  Value=KINP_LaneCrosL_flg_defval
  invalid : Constant  Value=KINP_LaneCrosL_flg_invalid
  ovrdflg : Constant  Value=KINP_LaneCrosL_flg_ovrdflg
  ovrdval : Constant  Value=KINP_LaneCrosL_flg_ovrdval
  setvalid : Constant  Value=KINP_LaneCrosL_flg_setvalid
  srvcflg : Constant  Value=KINP_LaneCrosL_flg_srvcflg
  srvcval : Constant  Value=KINP_LaneCrosL_flg_srvcval
  signal_out : Outport
  signal_valid_out : Outport  Port=2
  wires:
  Active_fault_flg/1 -> can_signal_process_wio_validity/3
  srvcval/1 -> can_signal_process_wio_validity/10
  srvcflg/1 -> can_signal_process_wio_validity/9
  ovrdval/1 -> can_signal_process_wio_validity/8
  ovrdflg/1 -> can_signal_process_wio_validity/7
  setvalid/1 -> can_signal_process_wio_validity/6
  invalid/1 -> can_signal_process_wio_validity/5
  defval/1 -> can_signal_process_wio_validity/4
  msg_valid/1 -> can_signal_process_wio_validity/2
  signal_in/1 -> can_signal_process_wio_validity/1
  can_signal_process_wio_validity/2 -> signal_valid_out/1
  can_signal_process_wio_validity/1 -> signal_out/1

== system inp/INP/EPM_signals/LaneCrossingRight ==
  signal_in : Inport
  msg_valid : Inport  Port=2
  Active_fault_flg : Inport  Port=3
  can_signal_process_wio_validity : inp/can_signal_process_wio_validity
  defval : Constant  Value=KINP_LaneCrosR_flg_defval
  invalid : Constant  Value=KINP_LaneCrosR_flg_invalid
  ovrdflg : Constant  Value=KINP_LaneCrosR_flg_ovrdflg
  ovrdval : Constant  Value=KINP_LaneCrosR_flg_ovrdval
  setvalid : Constant  Value=KINP_LaneCrosR_flg_setvalid
  srvcflg : Constant  Value=KINP_LaneCrosR_flg_srvcflg
  srvcval : Constant  Value=KINP_LaneCrosR_flg_srvcval
  signal_out : Outport
  signal_valid_out : Outport  Port=2
  wires:
  can_signal_process_wio_validity/1 -> signal_out/1
  can_signal_process_wio_validity/2 -> signal_valid_out/1
  signal_in/1 -> can_signal_process_wio_validity/1
  msg_valid/1 -> can_signal_process_wio_validity/2
  defval/1 -> can_signal_process_wio_validity/4
  invalid/1 -> can_signal_process_wio_validity/5
  setvalid/1 -> can_signal_process_wio_validity/6
  ovrdflg/1 -> can_signal_process_wio_validity/7
  ovrdval/1 -> can_signal_process_wio_validity/8
  srvcflg/1 -> can_signal_process_wio_validity/9
  srvcval/1 -> can_signal_process_wio_validity/10
  Active_fault_flg/1 -> can_signal_process_wio_validity/3

== system inp/INP/EPM_signals/LaneMarkColorLeft ==
  signal_in : Inport
  msg_valid : Inport  Port=2
  Active_fault_flg : Inport  Port=3
  can_signal_process_wio_validity : inp/can_signal_process_wio_validity
  defval : Constant  Value=KINP_LaneMrkClrL_enum_defval
  invalid : Constant  Value=KINP_LaneMrkClrL_enum_invalid
  ovrdflg : Constant  Value=KINP_LaneMrkClrL_enum_ovrdflg
  ovrdval : Constant  Value=KINP_LaneMrkClrL_enum_ovrdval
  setvalid : Constant  Value=KINP_LaneMrkClrL_enum_setvalid
  srvcflg : Constant  Value=KINP_LaneMrkClrL_enum_srvcflg
  srvcval : Constant  Value=KINP_LaneMrkClrL_enum_srvcval
  signal_out : Outport
  signal_valid_out : Outport  Port=2
  wires:
  can_signal_process_wio_validity/1 -> signal_out/1
  can_signal_process_wio_validity/2 -> signal_valid_out/1
  signal_in/1 -> can_signal_process_wio_validity/1
  msg_valid/1 -> can_signal_process_wio_validity/2
  defval/1 -> can_signal_process_wio_validity/4
  invalid/1 -> can_signal_process_wio_validity/5
  setvalid/1 -> can_signal_process_wio_validity/6
  ovrdflg/1 -> can_signal_process_wio_validity/7
  ovrdval/1 -> can_signal_process_wio_validity/8
  srvcflg/1 -> can_signal_process_wio_validity/9
  srvcval/1 -> can_signal_process_wio_validity/10
  Active_fault_flg/1 -> can_signal_process_wio_validity/3

== system inp/INP/EPM_signals/LaneMarkColorRight ==
  signal_in : Inport
  msg_valid : Inport  Port=2
  Active_fault_flg : Inport  Port=3
  can_signal_process_wio_validity : inp/can_signal_process_wio_validity
  defval : Constant  Value=KINP_LaneMrkClrR_enum_defval
  invalid : Constant  Value=KINP_LaneMrkClrR_enum_invalid
  ovrdflg : Constant  Value=KINP_LaneMrkClrR_enum_ovrdflg
  ovrdval : Constant  Value=KINP_LaneMrkClrR_enum_ovrdval
  setvalid : Constant  Value=KINP_LaneMrkClrR_enum_setvalid
  srvcflg : Constant  Value=KINP_LaneMrkClrR_enum_srvcflg
  srvcval : Constant  Value=KINP_LaneMrkClrR_enum_srvcval
  signal_out : Outport
  signal_valid_out : Outport  Port=2
  wires:
  Active_fault_flg/1 -> can_signal_process_wio_validity/3
  srvcval/1 -> can_signal_process_wio_validity/10
  srvcflg/1 -> can_signal_process_wio_validity/9
  ovrdval/1 -> can_signal_process_wio_validity/8
  ovrdflg/1 -> can_signal_process_wio_validity/7
  setvalid/1 -> can_signal_process_wio_validity/6
  invalid/1 -> can_signal_process_wio_validity/5
  defval/1 -> can_signal_process_wio_validity/4
  msg_valid/1 -> can_signal_process_wio_validity/2
  signal_in/1 -> can_signal_process_wio_validity/1
  can_signal_process_wio_validity/2 -> signal_valid_out/1
  can_signal_process_wio_validity/1 -> signal_out/1

== system inp/INP/EPM_signals/LaneMarkWidthLeft ==
  signal_in : Inport
  msg_valid : Inport  Port=2
  Active_fault_flg : Inport  Port=3
  can_signal_process_wio_validity : inp/can_signal_process_wio_validity
  conv_gain_offset : Subsystem
  defval : Constant  Value=KINP_LaneMrkWidL_m_defval
  invalid : Constant  Value=KINP_LaneMrkWidL_m_invalid
  min_max_limit : Subsystem
  ovrdflg : Constant  Value=KINP_LaneMrkWidL_m_ovrdflg
  ovrdval : Constant  Value=KINP_LaneMrkWidL_m_ovrdval
  setvalid : Constant  Value=KINP_LaneMrkWidL_m_setvalid
  srvcflg : Constant  Value=KINP_LaneMrkWidL_m_srvcflg
  srvcval : Constant  Value=KINP_LaneMrkWidL_m_srvcval
  signal_out : Outport
  signal_valid_out : Outport  Port=2
  wires:
  Active_fault_flg/1 -> can_signal_process_wio_validity/3
  srvcval/1 -> can_signal_process_wio_validity/10
  srvcflg/1 -> can_signal_process_wio_validity/9
  ovrdval/1 -> can_signal_process_wio_validity/8
  ovrdflg/1 -> can_signal_process_wio_validity/7
  setvalid/1 -> can_signal_process_wio_validity/6
  min_max_limit/1(out) -> signal_out/1
  conv_gain_offset/1(out) -> can_signal_process_wio_validity/1
  invalid/1 -> can_signal_process_wio_validity/5
  defval/1 -> can_signal_process_wio_validity/4
  msg_valid/1 -> can_signal_process_wio_validity/2
  signal_in/1 -> conv_gain_offset/1(in)
  can_signal_process_wio_validity/2 -> signal_valid_out/1
  can_signal_process_wio_validity/1 -> min_max_limit/1(in)

== system inp/INP/EPM_signals/LaneMarkWidthRight ==
  signal_in : Inport
  msg_valid : Inport  Port=2
  Active_fault_flg : Inport  Port=3
  can_signal_process_wio_validity : inp/can_signal_process_wio_validity
  conv_gain_offset : Subsystem
  defval : Constant  Value=KINP_LaneMrkWidR_m_defval
  invalid : Constant  Value=KINP_LaneMrkWidR_m_invalid
  min_max_limit : Subsystem
  ovrdflg : Constant  Value=KINP_LaneMrkWidR_m_ovrdflg
  ovrdval : Constant  Value=KINP_LaneMrkWidR_m_ovrdval
  setvalid : Constant  Value=KINP_LaneMrkWidR_m_setvalid
  srvcflg : Constant  Value=KINP_LaneMrkWidR_m_srvcflg
  srvcval : Constant  Value=KINP_LaneMrkWidR_m_srvcval
  signal_out : Outport
  signal_valid_out : Outport  Port=2
  wires:
  can_signal_process_wio_validity/1 -> min_max_limit/1(in)
  can_signal_process_wio_validity/2 -> signal_valid_out/1
  signal_in/1 -> conv_gain_offset/1(in)
  msg_valid/1 -> can_signal_process_wio_validity/2
  defval/1 -> can_signal_process_wio_validity/4
  invalid/1 -> can_signal_process_wio_validity/5
  conv_gain_offset/1(out) -> can_signal_process_wio_validity/1
  min_max_limit/1(out) -> signal_out/1
  setvalid/1 -> can_signal_process_wio_validity/6
  ovrdflg/1 -> can_signal_process_wio_validity/7
  ovrdval/1 -> can_signal_process_wio_validity/8
  srvcflg/1 -> can_signal_process_wio_validity/9
  srvcval/1 -> can_signal_process_wio_validity/10
  Active_fault_flg/1 -> can_signal_process_wio_validity/3

== system inp/INP/EPM_signals/LaneQualityLeft ==
  signal_in : Inport
  msg_valid : Inport  Port=2
  Active_fault_flg : Inport  Port=3
  can_signal_process_wio_validity : inp/can_signal_process_wio_validity
  defval : Constant  Value=KINP_LaneQualityL_enum_defval
  invalid : Constant  Value=KINP_LaneQualityL_enum_invalid
  ovrdflg : Constant  Value=KINP_LaneQualityL_enum_ovrdflg
  ovrdval : Constant  Value=KINP_LaneQualityL_enum_ovrdval
  setvalid : Constant  Value=KINP_LaneQualityL_enum_setvalid
  srvcflg : Constant  Value=KINP_LaneQualityL_enum_srvcflg
  srvcval : Constant  Value=KINP_LaneQualityL_enum_srvcval
  signal_out : Outport
  signal_valid_out : Outport  Port=2
  wires:
  can_signal_process_wio_validity/1 -> signal_out/1
  can_signal_process_wio_validity/2 -> signal_valid_out/1
  signal_in/1 -> can_signal_process_wio_validity/1
  msg_valid/1 -> can_signal_process_wio_validity/2
  defval/1 -> can_signal_process_wio_validity/4
  invalid/1 -> can_signal_process_wio_validity/5
  setvalid/1 -> can_signal_process_wio_validity/6
  ovrdflg/1 -> can_signal_process_wio_validity/7
  ovrdval/1 -> can_signal_process_wio_validity/8
  srvcflg/1 -> can_signal_process_wio_validity/9
  srvcval/1 -> can_signal_process_wio_validity/10
  Active_fault_flg/1 -> can_signal_process_wio_validity/3

== system inp/INP/EPM_signals/LaneQualityRight ==
  signal_in : Inport
  msg_valid : Inport  Port=2
  Active_fault_flg : Inport  Port=3
  can_signal_process_wio_validity : inp/can_signal_process_wio_validity
  defval : Constant  Value=KINP_LaneQualityR_enum_defval
  invalid : Constant  Value=KINP_LaneQualityR_enum_invalid
  ovrdflg : Constant  Value=KINP_LaneQualityR_enum_ovrdflg
  ovrdval : Constant  Value=KINP_LaneQualityR_enum_ovrdval
  setvalid : Constant  Value=KINP_LaneQualityR_enum_setvalid
  srvcflg : Constant  Value=KINP_LaneQualityR_enum_srvcflg
  srvcval : Constant  Value=KINP_LaneQualityR_enum_srvcval
  signal_out : Outport
  signal_valid_out : Outport  Port=2
  wires:
  Active_fault_flg/1 -> can_signal_process_wio_validity/3
  srvcval/1 -> can_signal_process_wio_validity/10
  srvcflg/1 -> can_signal_process_wio_validity/9
  ovrdval/1 -> can_signal_process_wio_validity/8
  ovrdflg/1 -> can_signal_process_wio_validity/7
  setvalid/1 -> can_signal_process_wio_validity/6
  invalid/1 -> can_signal_process_wio_validity/5
  defval/1 -> can_signal_process_wio_validity/4
  msg_valid/1 -> can_signal_process_wio_validity/2
  signal_in/1 -> can_signal_process_wio_validity/1
  can_signal_process_wio_validity/2 -> signal_valid_out/1
  can_signal_process_wio_validity/1 -> signal_out/1

== system inp/INP/EPM_signals/LaneTypeLeft ==
  signal_in : Inport
  msg_valid : Inport  Port=2
  Active_fault_flg : Inport  Port=3
  can_signal_process_wio_validity : inp/can_signal_process_wio_validity
  defval : Constant  Value=KINP_LaneTypeL_enum_defval
  invalid : Constant  Value=KINP_LaneTypeL_enum_invalid
  ovrdflg : Constant  Value=KINP_LaneTypeL_enum_ovrdflg
  ovrdval : Constant  Value=KINP_LaneTypeL_enum_ovrdval
  setvalid : Constant  Value=KINP_LaneTypeL_enum_setvalid
  srvcflg : Constant  Value=KINP_LaneTypeL_enum_srvcflg
  srvcval : Constant  Value=KINP_LaneTypeL_enum_srvcval
  signal_out : Outport
  signal_valid_out : Outport  Port=2
  wires:
  Active_fault_flg/1 -> can_signal_process_wio_validity/3
  srvcval/1 -> can_signal_process_wio_validity/10
  srvcflg/1 -> can_signal_process_wio_validity/9
  ovrdval/1 -> can_signal_process_wio_validity/8
  ovrdflg/1 -> can_signal_process_wio_validity/7
  setvalid/1 -> can_signal_process_wio_validity/6
  invalid/1 -> can_signal_process_wio_validity/5
  defval/1 -> can_signal_process_wio_validity/4
  msg_valid/1 -> can_signal_process_wio_validity/2
  signal_in/1 -> can_signal_process_wio_validity/1
  can_signal_process_wio_validity/2 -> signal_valid_out/1
  can_signal_process_wio_validity/1 -> signal_out/1

== system inp/INP/EPM_signals/LaneTypeRight ==
  signal_in : Inport
  msg_valid : Inport  Port=2
  Active_fault_flg : Inport  Port=3
  can_signal_process_wio_validity : inp/can_signal_process_wio_validity
  defval : Constant  Value=KINP_LaneTypeR_enum_defval
  invalid : Constant  Value=KINP_LaneTypeR_enum_invalid
  ovrdflg : Constant  Value=KINP_LaneTypeR_enum_ovrdflg
  ovrdval : Constant  Value=KINP_LaneTypeR_enum_ovrdval
  setvalid : Constant  Value=KINP_LaneTypeR_enum_setvalid
  srvcflg : Constant  Value=KINP_LaneTypeR_enum_srvcflg
  srvcval : Constant  Value=KINP_LaneTypeR_enum_srvcval
  signal_out : Outport
  signal_valid_out : Outport  Port=2
  wires:
  can_signal_process_wio_validity/1 -> signal_out/1
  can_signal_process_wio_validity/2 -> signal_valid_out/1
  signal_in/1 -> can_signal_process_wio_validity/1
  msg_valid/1 -> can_signal_process_wio_validity/2
  defval/1 -> can_signal_process_wio_validity/4
  invalid/1 -> can_signal_process_wio_validity/5
  setvalid/1 -> can_signal_process_wio_validity/6
  ovrdflg/1 -> can_signal_process_wio_validity/7
  ovrdval/1 -> can_signal_process_wio_validity/8
  srvcflg/1 -> can_signal_process_wio_validity/9
  srvcval/1 -> can_signal_process_wio_validity/10
  Active_fault_flg/1 -> can_signal_process_wio_validity/3

== system inp/INP/EPM_signals/TimeToLaneCrossingLeft ==
  signal_in : Inport
  msg_valid : Inport  Port=2
  Active_fault_flg : Inport  Port=3
  can_signal_process_wio_validity : inp/can_signal_process_wio_validity
  conv_gain_offset : Subsystem
  defval : Constant  Value=KINP_TLCL_s_defval
  invalid : Constant  Value=KINP_TLCL_s_invalid
  min_max_limit : Subsystem
  ovrdflg : Constant  Value=KINP_TLCL_s_ovrdflg
  ovrdval : Constant  Value=KINP_TLCL_s_ovrdval
  setvalid : Constant  Value=KINP_TLCL_s_setvalid
  srvcflg : Constant  Value=KINP_TLCL_s_srvcflg
  srvcval : Constant  Value=KINP_TLCL_s_srvcval
  signal_out : Outport
  signal_valid_out : Outport  Port=2
  wires:
  can_signal_process_wio_validity/1 -> min_max_limit/1(in)
  can_signal_process_wio_validity/2 -> signal_valid_out/1
  signal_in/1 -> conv_gain_offset/1(in)
  msg_valid/1 -> can_signal_process_wio_validity/2
  defval/1 -> can_signal_process_wio_validity/4
  invalid/1 -> can_signal_process_wio_validity/5
  conv_gain_offset/1(out) -> can_signal_process_wio_validity/1
  min_max_limit/1(out) -> signal_out/1
  setvalid/1 -> can_signal_process_wio_validity/6
  ovrdflg/1 -> can_signal_process_wio_validity/7
  ovrdval/1 -> can_signal_process_wio_validity/8
  srvcflg/1 -> can_signal_process_wio_validity/9
  srvcval/1 -> can_signal_process_wio_validity/10
  Active_fault_flg/1 -> can_signal_process_wio_validity/3
